simulink model "model"  (R2020b)
settings: Solver=FixedStepAuto  StartTime=0.0  StopTime=100  FixedStep=0.01  RelTol=1e-3
PreLoadFcn:
  | unit_step
settings Model: Creator=Arvind Hishikar  LastModifiedBy=Arvind Hishikar
settings DataIOCC: ExternalInput=[t, stin]  LoadExternalInput=on  SaveFormat=Array

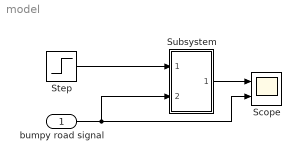
== system model ==
  bumpy road signal : Inport
  Scope : Scope  NumInputPorts=2
  Step : Step  SampleTime=0
  Subsystem : Subsystem
  wires:
  Subsystem/1(Out1) -> Scope/1
  Step/1 -> Subsystem/1(In1)
  bumpy road signal/1 -> Scope/2, Subsystem/2(In2)

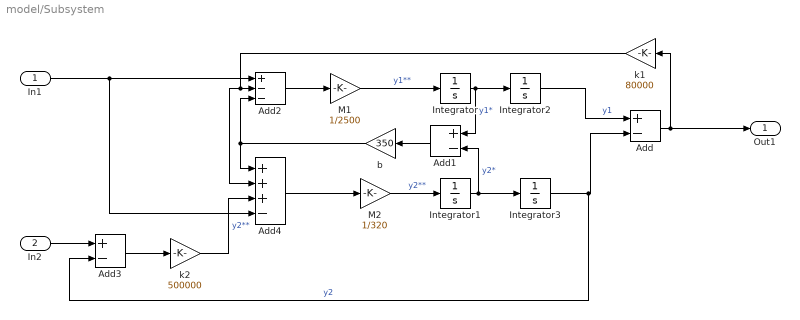
== system model/Subsystem ==
  In1 : Inport
  In2 : Inport  Port=2
  Add : Sum  Inputs=+-
  Add1 : Sum  Inputs=+-
  Add2 : Sum  Inputs=+--
  Add3 : Sum  Inputs=+-
  Add4 : Sum  Inputs=+++-
  Integrator{,1..3} (4 alike) : Integrator
  M1 : Gain  Gain=1/2500
  M2 : Gain  Gain=1/320
  b : Gain  Gain=350
  k1 : Gain  Gain=80000
  k2 : Gain  Gain=500000
  Out1 : Outport
  wires:
  b/1 -> Add2/3, Add4/1
  Integrator1/1 -> Add1/2, Integrator3/1  "y2*"
  Add3/1 -> k2/1
  Integrator3/1 -> Add3/2, Add/2  "y2"
  In1/1 -> Add4/4, Add2/1
  k1/1 -> Add4/2, Add2/2
  Add/1 -> k1/1, Out1/1
  k2/1 -> Add4/3  "y2**"
  M1/1 -> Integrator/1  "y1**"
  Integrator/1 -> Add1/1, Integrator2/1  "y1*"
  M2/1 -> Integrator1/1  "y2**"
  Integrator2/1 -> Add/1  "y1"
  Add1/1 -> b/1
  Add4/1 -> M2/1
  Add2/1 -> M1/1
  In2/1 -> Add3/1
